simulink model "mb4k-v155"  (R2013a)
settings: Solver=VariableStepDiscrete  SolverMode=SingleTasking  StartTime=0.0  StopTime=10^4  FixedStep=1  MaxStep=1  RelTol=1e-3
undefined symbols (not defined in the file): pfb_coeff_gen_calc, sfp
libraries: xbsIndex_r4 x28825, casper_library_misc x154, casper_library_delays x126, simulink x34, dspsigops x24, xbsReplacements_r4 x20, fast_library x16, xps_library x13, casper_library_sources x2
(part 1 of 293)
settings Model: Creator=zhuyan  LastModifiedBy=zhuyan
settings Model: ModifiedComment=|
  | zhuyan -- Mon Apr 09 13:55:30 2018 -- Version 1.155
  | update_casper_blocks('mb4k')
settings Model: ModifiedHistory=|
  | zhuyan -- Mon Apr 09 13:55:30 2018 -- Version 1.155
  | update_casper_blocks('mb4k')
  | 
  | zhuyan -- Sun Apr 08 15:18:23 2018 -- Version 1.154
  | minor: change sample time of 'step_reset' and 'step_arm' block from 0 to 1
  | 
  | zhuyan -- Tue Apr 03 14:44:45 2018 -- Version 1.151
  | extend noisecal registers to 48 bits each.
  | 
  | zhuyan -- Tue Apr 03 14:44:45 2018 -- Version 1.151
  | extend noisecal registers to 48 bits each.
  | 
  | zhuyan -- Mon Apr 02 14:41:34 2018 -- Version 1.150
  | Add "pps_counter"
  | 
  | zhuyan -- Thu Mar 29 14:54:53 2018 -- Version 1.149
  | change rcs_id to "mb4k"
  | 
  | zhuyan -- Fri Mar 23 14:29:22 2018 -- Version 1.147
  | increase ADC scope from 2048 to 4096 samples each  polarization
  | 
  | zhuyan -- Fri Mar 23 14:29:22 2018 -- Version 1.147
  | 
  | increase ADC scope from 2048 to 4096 samples per polarization
  | 
  | zhuyan -- Sun Jan 14 20:12:11 2018 -- Version 1.146
  | Disable fabric at startup in tenGbE_v2 blocks
  | 
  | zhuyan -- Thu Jan 11 19:18:11 2018 -- Version 1.145
  | correct typo.
  | replace wrong "From" label "sid_raw0" connected to pkt_raw1 to "sid_raw1".
  | 
  | zhuyan -- Sat Nov 11 22:55:54 2017 -- Version 1.144
  | bugfix: bit_select bits of cross term is the same as power term
settings OptimizationCC: InlineParams=on

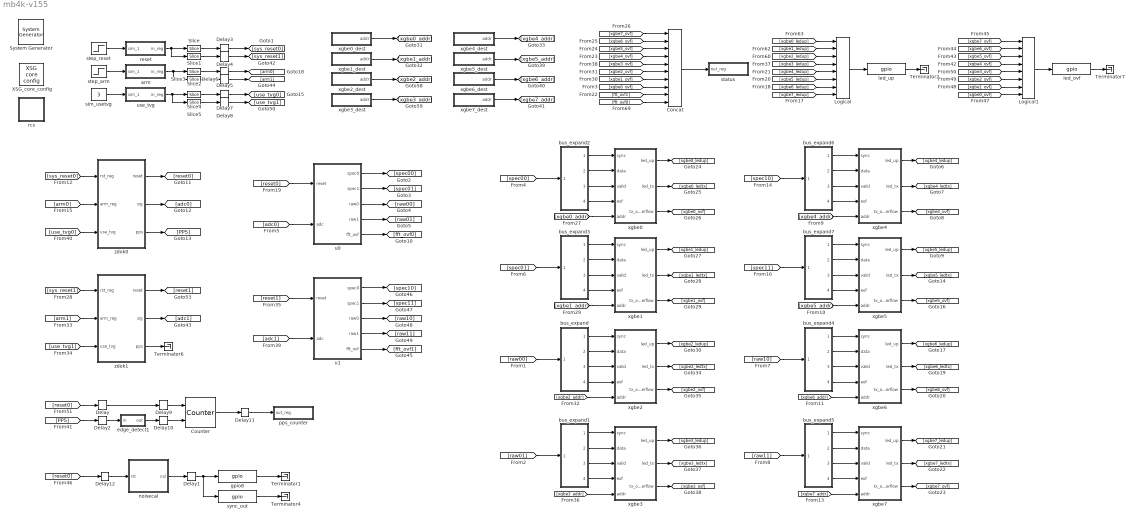
== system mb4k-v155 ==
  " System Generator" : xbsIndex_r4/ System Generator  Tag=genX  xilinxfamily=virtex6  part=xc6vsx475t  speed=-1  package=ff1759  synthesis_tool=XST  clock_wrapper=Clock Enables  directory=./mb4k/sysgen  proj_type=Project Navigator  Synth_file=XST Defaults  Impl_file=ISE Defaults  testbench=off  simulink_period=1  sysclk_period=10  dcm_input_clock_period=100  incr_netlist=off  trim_vbits=Everywhere in SubSystem  dbl_ovrd=According to Block Masks  core_generation=According to Block Masks  run_coregen=off  deprecated_control=off  eval_field=0  block_type=sysgen
  Concat : xbsIndex_r4/Concat  num_inputs=10  dbl_ovrd=off  block_type=concat
  Counter : xbsIndex_r4/Counter  cnt_type=Count Limited  cnt_to=Inf  operation=Up  start_count=0  cnt_by_val=1  arith_type=Unsigned  n_bits=32  bin_pt=0  load_pin=off  rst=on  en=on  explicit_period=on  period=1  dbl_ovrd=off  use_behavioral_HDL=on  implementation=Fabric  block_type=counter
  Delay{,1..12} (13 alike) : xbsIndex_r4/Delay  rst=off  infoeditControl=Selection of Reset will increase slice count due to use of real FFs and instead of SRLs  en=off  latency=1  dbl_ovrd=off  reg_retiming=off  block_type=delay
  Logical : xbsIndex_r4/Logical  logical_function=AND  inputs=8  en=off  latency=1  precision=Full  arith_type=Unsigned  n_bits=16  bin_pt=0  align_bp=on  dbl_ovrd=off  block_type=logical
  Logical1 : xbsIndex_r4/Logical  logical_function=OR  inputs=8  en=off  latency=1  precision=Full  arith_type=Unsigned  n_bits=16  bin_pt=0  align_bp=on  dbl_ovrd=off  block_type=logical
  Slice : xbsIndex_r4/Slice  nbits=1  boolean_output=on  mode=Lower Bit Location + Width  bit1=0  base1=MSB of Input  bit0=0  base0=LSB of Input  dbl_ovrd=off  block_type=slice
  Slice1 : xbsIndex_r4/Slice  nbits=1  boolean_output=on  mode=Lower Bit Location + Width  bit1=0  base1=MSB of Input  bit0=1  base0=LSB of Input  dbl_ovrd=off  block_type=slice
  Slice2 : xbsIndex_r4/Slice  nbits=1  boolean_output=on  mode=Lower Bit Location + Width  bit1=0  base1=MSB of Input  bit0=0  base0=LSB of Input  dbl_ovrd=off  block_type=slice
  Slice3 : xbsIndex_r4/Slice  nbits=1  boolean_output=on  mode=Lower Bit Location + Width  bit1=0  base1=MSB of Input  bit0=1  base0=LSB of Input  dbl_ovrd=off  block_type=slice
  Slice4 : xbsIndex_r4/Slice  nbits=1  boolean_output=on  mode=Lower Bit Location + Width  bit1=0  base1=MSB of Input  bit0=0  base0=LSB of Input  dbl_ovrd=off  block_type=slice
  Slice5 : xbsIndex_r4/Slice  nbits=1  boolean_output=on  mode=Lower Bit Location + Width  bit1=0  base1=MSB of Input  bit0=1  base0=LSB of Input  dbl_ovrd=off  block_type=slice
  Terminator{1..2,4,6..7} (5 alike) : Terminator
  XSG_core_config : xps_library/XSG core config  Tag=xps:xsg  hw_sys=ROACH2:sx475t  clk_src=adc0_clk  ROACH_clk_src=sys_clk  ROACH2_clk_src=adc0_clk  MKDIG_clk_src=sys_clk  clk_rate=100  sample_period=1  synthesis_tool=XST
  arm : Subsystem  Tag=xps:sw_reg  AncestorBlock=xps_library/software register  mask(swreg){io_dir=From Processor, io_delay=0, sample_period=1, names=reg, bitwidths=2, bin_pts=0, arith_types=0, sim_port=on, show_format=off}
      mask prompts: io_dir "I/O direction"; io_delay "I/O delay"; sample_period "Sample period"; names "Bitfield names [msb...lsb]"; bitwidths "Bitfield widths"; bin_pts "Bitfield binary pts"; arith_types "Bitfield types, ufix=0, fix=1, bool=2"; sim_port "Provide sim input/output?"; show_format "Print format string?"
      mask help: A 32-bit software-accessible register. Can be divided into bitfields of varying widths and types using the fields in the block mask.
      mask init:
      | swreg_init(gcb)
      settings: AttributesFormatString=1input
      mask TypeOptions io_dir: To Processor | From Processor
      mask Callback io_dir:
      | mask_enables = get_param(gcb, 'MaskEnables');
      | mask_names = get_param(gcb, 'MaskNames');
      | if strcmp(get_param(gcb, 'io_dir'), 'To Processor'),
      |     mask_enables{ismember(mask_names, 'sample_period')} = 'off';
      | else
      |     mask_enables{ismember(mask_names, 'sample_period')} = 'on';
      | end
      | set_param(gcb, 'MaskEnables', mask_enables);
  bus_expand : Subsystem  AncestorBlock=casper_library_flow_control/bus_expand  mask(bus_expand){mode=divisions of arbitrary size, outputNum=1, outputWidth=[1 64 1 1], outputBinaryPt=0, outputArithmeticType=[2 0 2 2], show_format=off, outputToWorkspace=off, variablePrefix=out, outputToModelAsWell=on}
      mask prompts: outputNum "Number of outputs:"; outputWidth "Output width:"; outputBinaryPt "Output binary point position:"; outputArithmeticType "Output arithmetic type (ufix=0, fix=1, bool=2):"; show_format "Print format string?"; outputToWorkspace "Output to workspace?"; variablePrefix "Variable name prefix:"; outputToModelAsWell "Output to model as well?"
      mask help:
      | Expands a 'bus' made using a bus_create or Xilinx
      | concat block.
      | The input will be divided into the specified number
      | of outputs with the specified arithmetic types.
      | Divisions are made from most significant bit.
      mask init:
      | bus_expand_init(gcb,...
      |     'mode', mode, ...
      |     'outputNum', outputNum, ...
      |     'outputWidth', outputWidth, ...
      |     'outputBinaryPt', outputBinaryPt, ...
      |     'outputArithmeticType', outputArithmeticType, ...
      |     'show_format', show_format, ...
      |     'outputToWorkspace', outputToWorkspace, ...
      |     'variablePrefix', variablePrefix, ...
      |     'outputToModelAsWell', outputToModelAsWell);
      settings: AttributesFormatString=4 outputs
      mask TypeOptions mode: divisions of equal size | divisions of arbitrary size
      mask Callback mode: bus_expand_callback();
      mask Callback outputWidth: bus_expand_callback();
      mask Callback outputToWorkspace: bus_expand_callback();
  bus_expand1 : Subsystem  AncestorBlock=casper_library_flow_control/bus_expand  mask(bus_expand){mode=divisions of arbitrary size, outputNum=1, outputWidth=[1 64 1 1], outputBinaryPt=0, outputArithmeticType=[2 0 2 2], show_format=off, outputToWorkspace=off, variablePrefix=out, outputToModelAsWell=on}
      mask prompts: outputNum "Number of outputs:"; outputWidth "Output width:"; outputBinaryPt "Output binary point position:"; outputArithmeticType "Output arithmetic type (ufix=0, fix=1, bool=2):"; show_format "Print format string?"; outputToWorkspace "Output to workspace?"; variablePrefix "Variable name prefix:"; outputToModelAsWell "Output to model as well?"
      mask help:
      | Expands a 'bus' made using a bus_create or Xilinx
      | concat block.
      | The input will be divided into the specified number
      | of outputs with the specified arithmetic types.
      | Divisions are made from most significant bit.
      mask init: same as mb4k-v155/bus_expand
      settings: AttributesFormatString=4 outputs
      mask TypeOptions mode: divisions of equal size | divisions of arbitrary size
      mask Callback mode: bus_expand_callback();
      mask Callback outputWidth: bus_expand_callback();
      mask Callback outputToWorkspace: bus_expand_callback();
  bus_expand2 : Subsystem  AncestorBlock=casper_library_flow_control/bus_expand  mask(bus_expand){mode=divisions of arbitrary size, outputNum=1, outputWidth=[1 64 1 1], outputBinaryPt=0, outputArithmeticType=[2 0 2 2], show_format=off, outputToWorkspace=off, variablePrefix=out, outputToModelAsWell=on}
      mask prompts: outputNum "Number of outputs:"; outputWidth "Output width:"; outputBinaryPt "Output binary point position:"; outputArithmeticType "Output arithmetic type (ufix=0, fix=1, bool=2):"; show_format "Print format string?"; outputToWorkspace "Output to workspace?"; variablePrefix "Variable name prefix:"; outputToModelAsWell "Output to model as well?"
      mask help:
      | Expands a 'bus' made using a bus_create or Xilinx
      | concat block.
      | The input will be divided into the specified number
      | of outputs with the specified arithmetic types.
      | Divisions are made from most significant bit.
      mask init: same as mb4k-v155/bus_expand
      settings: AttributesFormatString=4 outputs
      mask TypeOptions mode: divisions of equal size | divisions of arbitrary size
      mask Callback mode: bus_expand_callback();
      mask Callback outputWidth: bus_expand_callback();
      mask Callback outputToWorkspace: bus_expand_callback();
  bus_expand3 : Subsystem  AncestorBlock=casper_library_flow_control/bus_expand  mask(bus_expand){mode=divisions of arbitrary size, outputNum=1, outputWidth=[1 64 1 1], outputBinaryPt=0, outputArithmeticType=[2 0 2 2], show_format=off, outputToWorkspace=off, variablePrefix=out, outputToModelAsWell=on}
      mask prompts: outputNum "Number of outputs:"; outputWidth "Output width:"; outputBinaryPt "Output binary point position:"; outputArithmeticType "Output arithmetic type (ufix=0, fix=1, bool=2):"; show_format "Print format string?"; outputToWorkspace "Output to workspace?"; variablePrefix "Variable name prefix:"; outputToModelAsWell "Output to model as well?"
      mask help:
      | Expands a 'bus' made using a bus_create or Xilinx
      | concat block.
      | The input will be divided into the specified number
      | of outputs with the specified arithmetic types.
      | Divisions are made from most significant bit.
      mask init: same as mb4k-v155/bus_expand
      settings: AttributesFormatString=4 outputs
      mask TypeOptions mode: divisions of equal size | divisions of arbitrary size
      mask Callback mode: bus_expand_callback();
      mask Callback outputWidth: bus_expand_callback();
      mask Callback outputToWorkspace: bus_expand_callback();
  bus_expand4 : Subsystem  AncestorBlock=casper_library_flow_control/bus_expand  mask(bus_expand){mode=divisions of arbitrary size, outputNum=1, outputWidth=[1 64 1 1], outputBinaryPt=0, outputArithmeticType=[2 0 2 2], show_format=off, outputToWorkspace=off, variablePrefix=out, outputToModelAsWell=on}
      mask prompts: outputNum "Number of outputs:"; outputWidth "Output width:"; outputBinaryPt "Output binary point position:"; outputArithmeticType "Output arithmetic type (ufix=0, fix=1, bool=2):"; show_format "Print format string?"; outputToWorkspace "Output to workspace?"; variablePrefix "Variable name prefix:"; outputToModelAsWell "Output to model as well?"
      mask help:
      | Expands a 'bus' made using a bus_create or Xilinx
      | concat block.
      | The input will be divided into the specified number
      | of outputs with the specified arithmetic types.
      | Divisions are made from most significant bit.
      mask init: same as mb4k-v155/bus_expand
      settings: AttributesFormatString=4 outputs
      mask TypeOptions mode: divisions of equal size | divisions of arbitrary size
      mask Callback mode: bus_expand_callback();
      mask Callback outputWidth: bus_expand_callback();
      mask Callback outputToWorkspace: bus_expand_callback();
  bus_expand5 : Subsystem  AncestorBlock=casper_library_flow_control/bus_expand  mask(bus_expand){mode=divisions of arbitrary size, outputNum=1, outputWidth=[1 64 1 1], outputBinaryPt=0, outputArithmeticType=[2 0 2 2], show_format=off, outputToWorkspace=off, variablePrefix=out, outputToModelAsWell=on}
      mask prompts: outputNum "Number of outputs:"; outputWidth "Output width:"; outputBinaryPt "Output binary point position:"; outputArithmeticType "Output arithmetic type (ufix=0, fix=1, bool=2):"; show_format "Print format string?"; outputToWorkspace "Output to workspace?"; variablePrefix "Variable name prefix:"; outputToModelAsWell "Output to model as well?"
      mask help:
      | Expands a 'bus' made using a bus_create or Xilinx
      | concat block.
      | The input will be divided into the specified number
      | of outputs with the specified arithmetic types.
      | Divisions are made from most significant bit.
      mask init: same as mb4k-v155/bus_expand
      settings: AttributesFormatString=4 outputs
      mask TypeOptions mode: divisions of equal size | divisions of arbitrary size
      mask Callback mode: bus_expand_callback();
      mask Callback outputWidth: bus_expand_callback();
      mask Callback outputToWorkspace: bus_expand_callback();
  bus_expand6 : Subsystem  AncestorBlock=casper_library_flow_control/bus_expand  mask(bus_expand){mode=divisions of arbitrary size, outputNum=1, outputWidth=[1 64 1 1], outputBinaryPt=0, outputArithmeticType=[2 0 2 2], show_format=off, outputToWorkspace=off, variablePrefix=out, outputToModelAsWell=on}
      mask prompts: outputNum "Number of outputs:"; outputWidth "Output width:"; outputBinaryPt "Output binary point position:"; outputArithmeticType "Output arithmetic type (ufix=0, fix=1, bool=2):"; show_format "Print format string?"; outputToWorkspace "Output to workspace?"; variablePrefix "Variable name prefix:"; outputToModelAsWell "Output to model as well?"
      mask help:
      | Expands a 'bus' made using a bus_create or Xilinx
      | concat block.
      | The input will be divided into the specified number
      | of outputs with the specified arithmetic types.
      | Divisions are made from most significant bit.
      mask init: same as mb4k-v155/bus_expand
      settings: AttributesFormatString=4 outputs
      mask TypeOptions mode: divisions of equal size | divisions of arbitrary size
      mask Callback mode: bus_expand_callback();
      mask Callback outputWidth: bus_expand_callback();
      mask Callback outputToWorkspace: bus_expand_callback();
  bus_expand7 : Subsystem  AncestorBlock=casper_library_flow_control/bus_expand  mask(bus_expand){mode=divisions of arbitrary size, outputNum=1, outputWidth=[1 64 1 1], outputBinaryPt=0, outputArithmeticType=[2 0 2 2], show_format=off, outputToWorkspace=off, variablePrefix=out, outputToModelAsWell=on}
      mask prompts: outputNum "Number of outputs:"; outputWidth "Output width:"; outputBinaryPt "Output binary point position:"; outputArithmeticType "Output arithmetic type (ufix=0, fix=1, bool=2):"; show_format "Print format string?"; outputToWorkspace "Output to workspace?"; variablePrefix "Variable name prefix:"; outputToModelAsWell "Output to model as well?"
      mask help:
      | Expands a 'bus' made using a bus_create or Xilinx
      | concat block.
      | The input will be divided into the specified number
      | of outputs with the specified arithmetic types.
      | Divisions are made from most significant bit.
      mask init: same as mb4k-v155/bus_expand
      settings: AttributesFormatString=4 outputs
      mask TypeOptions mode: divisions of equal size | divisions of arbitrary size
      mask Callback mode: bus_expand_callback();
      mask Callback outputWidth: bus_expand_callback();
      mask Callback outputToWorkspace: bus_expand_callback();
  edge_detect1 : Subsystem  AncestorBlock=casper_library_misc/edge_detect  mask(edge_detect){edge=Rising, polarity=Active High, x_in=[0 0.08 0.08 0.16 0.16 0.24 0.24 0.32 0.32 0.4], y_in=[-1 -1  1  1  1  1  1  1  1  1], x_out=[0.6 0.68 0.68 0.76 0.76 0.84 0.84 0.92 0.92 1], y_out=[-1 -1  1  1 -1 -1 -1 -1 -1 -1]}
      mask prompts: edge "Edge Type"; polarity "Output Polarity"; x_in "Input X Positions"; y_in "Input Y Positions"; x_out "Output X Positions"; y_out "Output Y Positions"
      mask help: Output is active for one-cycle after each edge of the specified type (i.e. rising, falling or both).
      mask init:
      | edge_detect_init(gcb,...
      |     'edge',edge,...
      |     'polarity',polarity,...
      |     'x_in',x_in,...
      |     'x_out',x_out,...
      |     'y_in',y_in,...
      |     'y_out',y_out);
      | 
      | x_in_num=str2num(getfield(getfield(get_param(gcb,'UserData'),'parameters'),'x_in'));
      | x_out_num=str2num(getfield(getfield(get_param(gcb,'UserData'),'parameters'),'x_out'));
      | y_in_num=str2num(getfield(getfield(get_param(gcb,'UserData'),'parameters'),'y_in'));
      | y_out_num=str2num(getfield(getfield(get_param(gcb,'UserData'),'parameters'),'y_out'));
      settings: AttributesFormatString=|
      | rising edges
      | active high
      mask Help: Output is active for one-cycle after each edge of the specified type (i.e. rising, falling, or both).  Latency is zero.
      mask TypeOptions edge: Rising | Falling | Both
      mask TypeOptions polarity: Active High | Active Low
      mask Display:
      | plot(x_in_num,0.5+y_in_num*0.2);
      | plot(x_out_num,0.5+y_out_num*0.2);
  gpio8 : xps_library/gpio  Tag=xps:gpio  io_group=ROACH2:gpio  io_dir=out  arith_type=Boolean  bitwidth=1  bin_pt=0  bit_index=0  sample_period=1  use_single_ended=off  use_ddr=off  reg_iob=on  reg_clk_phase=0  termination=None
  led_ovf : xps_library/gpio  Tag=xps:gpio  io_group=ROACH2:led  io_dir=out  arith_type=Boolean  bitwidth=1  bin_pt=0  bit_index=4  sample_period=1  use_single_ended=off  use_ddr=off  reg_iob=on  reg_clk_phase=0  termination=None
  led_up : xps_library/gpio  Tag=xps:gpio  io_group=ROACH2:led  io_dir=out  arith_type=Boolean  bitwidth=1  bin_pt=0  bit_index=0  sample_period=1  use_single_ended=off  use_ddr=off  reg_iob=on  reg_clk_phase=0  termination=None
  noisecal : Subsystem  mask(noisecal){}
      mask help:
      | Square wave to control external noise source.
      |
      | delay - number of clocks before the noise on signal after reset+latency
      | delay_hipart - higher 16 bits of "delay" register, total 48 bits
      | on - number of clocks in on state
      | on_hipart - higher 16 bits of "on" register, total 48 bits
      | off -number of clocks in off state
      | off_hipart - higher 16 bits of "off" register, total 48 bits
      |
      | sequence:
      | reset->[latency]->delay->on->off->on->off->...
      mask Display:
      | port_label('input', 1, 'rst');
      | port_label('output', 1, 'cal');
      | disp('z^{-5}','texmode','on');
  pps_counter : Subsystem  Tag=xps:sw_reg  AncestorBlock=xps_library/software register  mask(swreg){io_dir=To Processor, io_delay=0, sample_period=1, names=reg, bitwidths=32, bin_pts=0, arith_types=0, sim_port=off, show_format=off}
      mask prompts: io_dir "I/O direction"; io_delay "I/O delay"; sample_period "Sample period"; names "Bitfield names [msb...lsb]"; bitwidths "Bitfield widths"; bin_pts "Bitfield binary pts"; arith_types "Bitfield types, ufix=0, fix=1, bool=2"; sim_port "Provide sim input/output?"; show_format "Print format string?"
      mask help: A 32-bit software-accessible register. Can be divided into bitfields of varying widths and types using the fields in the block mask.
      mask init:
      | swreg_init(gcb)
      settings: AttributesFormatString=1output
      mask TypeOptions io_dir: To Processor | From Processor
      mask Callback io_dir:
      | mask_enables = get_param(gcb, 'MaskEnables');
      | mask_names = get_param(gcb, 'MaskNames');
      | if strcmp(get_param(gcb, 'io_dir'), 'To Processor'),
      |     mask_enables{ismember(mask_names, 'sample_period')} = 'off';
      | else
      |     mask_enables{ismember(mask_names, 'sample_period')} = 'on';
      | end
      | set_param(gcb, 'MaskEnables', mask_enables);
  rcs : Subsystem  AncestorBlock=fast_library/myrcs  mask(myrcs){proj_id=mb4k}
      mask prompts: proj_id "Project ID:"
      mask help:
      | Revision control block.
      |
      | proj_id - exact 4 characters filled by user.
      | timestamp - seconds from unix epoch.
      | version - high 16 bits are major and low 16 bits are minor.
      |
      | The timestamp and version are taken from model properties.
      mask init:
      | blk = gcb();
      | sys = bdroot();
      | 
      | if length(proj_id) ~= 4
      |     error('Project ID must be exact 4 characters');
      | end
      | 
      | set_param(blk, 'LinkStatus', 'inactive')
      | 
      | rcs_id = proj_id(1)*256^3 + proj_id(2)*256^2 + proj_id(3)*256 + proj_id(4);
      | set_param([blk '/rcs_id'], 'const', num2str(rcs_id));
      | 
      | unix_epoch = datenum(1970, 1, 1, 0, 0, 0);
      | mdate = datenum(get_param(sys, 'LastModifiedDate'), 'ddd mmm dd HH:MM:SS yyyy');
      | unix_time = round((mdate - unix_epoch) * 24*60*60);
      | set_param([blk '/rcs_timestamp'], 'const', num2str(unix_time));
      | 
      | vers = sscanf(get_param(sys, 'ModelVersion'), '%d.%d');
      | rcs_ver = num2str(vers(1)*65536 + vers(2));
      | set_param([blk '/rcs_ver'], 'const', rcs_ver);
      mask Display: fprintf(proj_id);
  reset : Subsystem  Tag=xps:sw_reg  AncestorBlock=xps_library/software register  mask(swreg){io_dir=From Processor, io_delay=0, sample_period=1, names=reg, bitwidths=2, bin_pts=0, arith_types=0, sim_port=on, show_format=off}
      mask prompts: io_dir "I/O direction"; io_delay "I/O delay"; sample_period "Sample period"; names "Bitfield names [msb...lsb]"; bitwidths "Bitfield widths"; bin_pts "Bitfield binary pts"; arith_types "Bitfield types, ufix=0, fix=1, bool=2"; sim_port "Provide sim input/output?"; show_format "Print format string?"
      mask help: A 32-bit software-accessible register. Can be divided into bitfields of varying widths and types using the fields in the block mask.
      mask init:
      | swreg_init(gcb)
      settings: AttributesFormatString=1input
      mask TypeOptions io_dir: To Processor | From Processor
      mask Callback io_dir:
      | mask_enables = get_param(gcb, 'MaskEnables');
      | mask_names = get_param(gcb, 'MaskNames');
      | if strcmp(get_param(gcb, 'io_dir'), 'To Processor'),
      |     mask_enables{ismember(mask_names, 'sample_period')} = 'off';
      | else
      |     mask_enables{ismember(mask_names, 'sample_period')} = 'on';
      | end
      | set_param(gcb, 'MaskEnables', mask_enables);
  sim_usetvg : Constant  Value=3
  status : Subsystem  Tag=xps:sw_reg  AncestorBlock=xps_library/software register  mask(swreg){io_dir=To Processor, io_delay=0, sample_period=1, names=reg, bitwidths=12, bin_pts=0, arith_types=0, sim_port=off, show_format=off}
      mask prompts: io_dir "I/O direction"; io_delay "I/O delay"; sample_period "Sample period"; names "Bitfield names [msb...lsb]"; bitwidths "Bitfield widths"; bin_pts "Bitfield binary pts"; arith_types "Bitfield types, ufix=0, fix=1, bool=2"; sim_port "Provide sim input/output?"; show_format "Print format string?"
      mask help: A 32-bit software-accessible register. Can be divided into bitfields of varying widths and types using the fields in the block mask.
      mask init:
      | swreg_init(gcb)
      settings: AttributesFormatString=1output
      mask TypeOptions io_dir: To Processor | From Processor
      mask Callback io_dir:
      | mask_enables = get_param(gcb, 'MaskEnables');
      | mask_names = get_param(gcb, 'MaskNames');
      | if strcmp(get_param(gcb, 'io_dir'), 'To Processor'),
      |     mask_enables{ismember(mask_names, 'sample_period')} = 'off';
      | else
      |     mask_enables{ismember(mask_names, 'sample_period')} = 'on';
      | end
      | set_param(gcb, 'MaskEnables', mask_enables);
  step_arm : Step  After=7  SampleTime=1
  step_reset : Step  SampleTime=1
  sync_out : xps_library/gpio  Tag=xps:gpio  io_group=ROACH2:sync_out  io_dir=out  arith_type=Boolean  bitwidth=1  bin_pt=0  bit_index=0  sample_period=1  use_single_ended=off  use_ddr=off  reg_iob=on  reg_clk_phase=0  termination=Pulldown
  u0 : Subsystem  mask{nbins=4096}
      mask prompts: nbins "Num frequency channels:"
      mask init:
      | binbits = nextpow2(str2num(get_param(gcb, 'nbins')));
      | old_fftbits = str2num(get_param([gcb, '/x4_spec'], 'FFTSize'));
      | if binbits + 1 == old_fftbits
      |     return
      | end
      | 
      | blk = [gcb, '/x4_spec'];
      | param = 'FFTSize';
      | oldval = str2num(get_param(blk, param));
      | newval = binbits + 1;
      | if oldval ~= newval
      |     set_param(blk, param, num2str(newval));
      | end
      | 
      | blk = [gcb, '/x4_vacc'];
      | param = 'bin_bits';
      | oldval = str2num(get_param(blk, param));
      | newval = binbits;
      | if oldval ~= newval
      |     set_param(blk, param, num2str(newval));
      | end
      | oldval = get_param(blk, param);
      | 
      | blk = [gcb, '/pkt_vacc0'];
      | param = 'nbits';
      | oldval = str2num(get_param(blk, param));
      | newval = binbits - 1;
      | if newval > 10
      |     newval = 10;
      | end
      | if oldval ~= newval
      |     set_param(blk, param, num2str(newval));
      | end
      | 
      | blk = [gcb, '/pkt_vacc1'];
      | param = 'nbits';
      | oldval = str2num(get_param(blk, param));
      | if oldval ~= newval
      |     set_param(blk, param, num2str(newval));
      | end
      mask TypeOptions nbins: 1024 | 2048 | 4096 | 8192
  u1 : Subsystem  mask{nbins=4096}
      mask prompts: nbins "Num frequency channels:"
      mask init: same as mb4k-v155/u0
      mask TypeOptions nbins: 1024 | 2048 | 4096 | 8192
  use_tvg : Subsystem  Tag=xps:sw_reg  AncestorBlock=xps_library/software register  mask(swreg){io_dir=From Processor, io_delay=0, sample_period=1, names=reg, bitwidths=2, bin_pts=0, arith_types=0, sim_port=on, show_format=off}
      mask prompts: io_dir "I/O direction"; io_delay "I/O delay"; sample_period "Sample period"; names "Bitfield names [msb...lsb]"; bitwidths "Bitfield widths"; bin_pts "Bitfield binary pts"; arith_types "Bitfield types, ufix=0, fix=1, bool=2"; sim_port "Provide sim input/output?"; show_format "Print format string?"
      mask help: A 32-bit software-accessible register. Can be divided into bitfields of varying widths and types using the fields in the block mask.
      mask init:
      | swreg_init(gcb)
      settings: AttributesFormatString=1input
      mask TypeOptions io_dir: To Processor | From Processor
      mask Callback io_dir:
      | mask_enables = get_param(gcb, 'MaskEnables');
      | mask_names = get_param(gcb, 'MaskNames');
      | if strcmp(get_param(gcb, 'io_dir'), 'To Processor'),
      |     mask_enables{ismember(mask_names, 'sample_period')} = 'off';
      | else
      |     mask_enables{ismember(mask_names, 'sample_period')} = 'on';
      | end
      | set_param(gcb, 'MaskEnables', mask_enables);
  xgbe0 : Subsystem
  xgbe0_dest : Subsystem
  xgbe1 : Subsystem
  xgbe1_dest : Subsystem
  xgbe2 : Subsystem
  xgbe2_dest : Subsystem
  xgbe3 : Subsystem
  xgbe3_dest : Subsystem
  xgbe4 : Subsystem
  xgbe4_dest : Subsystem
  xgbe5 : Subsystem
  xgbe5_dest : Subsystem
  xgbe6 : Subsystem
  xgbe6_dest : Subsystem
  xgbe7 : Subsystem
  xgbe7_dest : Subsystem
  zdok0 : Subsystem  mask{adc_clk_rate=1024}
      mask prompts: adc_clk_rate "ADC clock rate (MHz)"
  zdok1 : Subsystem  mask{adc_clk_rate=1024}
      mask prompts: adc_clk_rate "ADC clock rate (MHz)"
  wires:
  step_reset/1 -> reset/1(sim_1)
  reset/1(in_reg) -> Slice1/1, Slice/1
  sim_usetvg/1 -> use_tvg/1(sim_1)
  use_tvg/1(in_reg) -> Slice4/1, Slice5/1
  step_arm/1 -> arm/1(sim_1)
  arm/1(in_reg) -> Slice3/1, Slice2/1
  [use_tvg0] -> zdok0/3(use_tvg)
  [arm0] -> zdok0/2(arm_reg)
  [sys_reset0] -> zdok0/1(rst_reg)
  zdok0/3(pps) -> [PPS]
  zdok0/1(reset) -> [reset0]
  zdok0/2(sig) -> [adc0]
  xgbe0/1(led_up) -> [xgbe0_ledup]
  xgbe0/2(led_tx) -> [xgbe0_ledtx]
  xgbe0/3(tx_overflow) -> [xgbe0_ovf]
  xgbe1/1(led_up) -> [xgbe1_ledup]
  xgbe1/2(led_tx) -> [xgbe1_ledtx]
  xgbe1/3(tx_overflow) -> [xgbe1_ovf]
  xgbe0_dest/1(addr) -> [xgbe0_addr]
  xgbe1_dest/1(addr) -> [xgbe1_addr]
  [xgbe0_addr] -> xgbe0/5(addr)
  [xgbe1_addr] -> xgbe1/5(addr)
  Logical/1 -> led_up/1
  led_up/1 -> Terminator2/1
  Concat/1 -> status/1(out_reg)
  bus_expand/1(msb_out4) -> xgbe2/1(sync)
  bus_expand/2(out3) -> xgbe2/2(data)
  bus_expand/3(out2) -> xgbe2/3(valid)
  bus_expand/4(lsb_out1) -> xgbe2/4(eof)
  [xgbe2_addr] -> xgbe2/5(addr)
  xgbe2/1(led_up) -> [xgbe2_ledup]
  xgbe2/2(led_tx) -> [xgbe2_ledtx]
  xgbe2/3(tx_overflow) -> [xgbe2_ovf]
  [xgbe3_addr] -> xgbe3/5(addr)
  xgbe3/1(led_up) -> [xgbe3_ledup]
  xgbe3/2(led_tx) -> [xgbe3_ledtx]
  xgbe3/3(tx_overflow) -> [xgbe3_ovf]
  xgbe2_dest/1(addr) -> [xgbe2_addr]
  xgbe3_dest/1(addr) -> [xgbe3_addr]
  [xgbe1_ledup] -> Logical/2
  [xgbe2_ledup] -> Logical/3
  [xgbe0_ledup] -> Logical/1
  [xgbe3_ledup] -> Logical/4
  bus_expand2/1(msb_out4) -> xgbe0/1(sync)
  bus_expand3/1(msb_out4) -> xgbe1/1(sync)
  bus_expand2/2(out3) -> xgbe0/2(data)
  bus_expand2/3(out2) -> xgbe0/3(valid)
  bus_expand2/4(lsb_out1) -> xgbe0/4(eof)
  bus_expand3/3(out2) -> xgbe1/3(valid)
  bus_expand3/4(lsb_out1) -> xgbe1/4(eof)
  bus_expand3/2(out3) -> xgbe1/2(data)
  [reset0] -> u0/1(reset)
  [adc0] -> u0/2(adc)
  u0/1(spec0) -> [spec00]
  [raw00] -> bus_expand/1(bus_in)
  [raw01] -> bus_expand1/1(bus_in)
  bus_expand1/1(msb_out4) -> xgbe3/1(sync)
  bus_expand1/2(out3) -> xgbe3/2(data)
  bus_expand1/3(out2) -> xgbe3/3(valid)
  bus_expand1/4(lsb_out1) -> xgbe3/4(eof)
  [spec00] -> bus_expand2/1(bus_in)
  [spec01] -> bus_expand3/1(bus_in)
  u0/2(spec1) -> [spec01]
  u0/3(raw0) -> [raw00]
  u0/4(raw1) -> [raw01]
  u0/5(fft_ovf) -> [fft_ovf0]
  xgbe4/1(led_up) -> [xgbe4_ledup]
  xgbe4/2(led_tx) -> [xgbe4_ledtx]
  xgbe4/3(tx_overflow) -> [xgbe4_ovf]
  xgbe5/1(led_up) -> [xgbe5_ledup]
  xgbe5/2(led_tx) -> [xgbe5_ledtx]
  xgbe5/3(tx_overflow) -> [xgbe5_ovf]
  [xgbe4_addr] -> xgbe4/5(addr)
  [xgbe5_addr] -> xgbe5/5(addr)
  bus_expand4/1(msb_out4) -> xgbe6/1(sync)
  bus_expand4/2(out3) -> xgbe6/2(data)
  bus_expand4/3(out2) -> xgbe6/3(valid)
  bus_expand4/4(lsb_out1) -> xgbe6/4(eof)
  [xgbe6_addr] -> xgbe6/5(addr)
  xgbe6/1(led_up) -> [xgbe6_ledup]
  xgbe6/2(led_tx) -> [xgbe6_ledtx]
  xgbe6/3(tx_overflow) -> [xgbe6_ovf]
  [xgbe7_addr] -> xgbe7/5(addr)
  xgbe7/1(led_up) -> [xgbe7_ledup]
  xgbe7/2(led_tx) -> [xgbe7_ledtx]
  xgbe7/3(tx_overflow) -> [xgbe7_ovf]
  bus_expand6/1(msb_out4) -> xgbe4/1(sync)
  bus_expand7/1(msb_out4) -> xgbe5/1(sync)
  bus_expand6/2(out3) -> xgbe4/2(data)
  bus_expand6/3(out2) -> xgbe4/3(valid)
  bus_expand6/4(lsb_out1) -> xgbe4/4(eof)
  bus_expand7/3(out2) -> xgbe5/3(valid)
  bus_expand7/4(lsb_out1) -> xgbe5/4(eof)
  bus_expand7/2(out3) -> xgbe5/2(data)
  [raw10] -> bus_expand4/1(bus_in)
  [raw11] -> bus_expand5/1(bus_in)
  bus_expand5/1(msb_out4) -> xgbe7/1(sync)
  bus_expand5/2(out3) -> xgbe7/2(data)
  bus_expand5/3(out2) -> xgbe7/3(valid)
  bus_expand5/4(lsb_out1) -> xgbe7/4(eof)
  [spec10] -> bus_expand6/1(bus_in)
  [spec11] -> bus_expand7/1(bus_in)
  xgbe4_dest/1(addr) -> [xgbe4_addr]
  xgbe5_dest/1(addr) -> [xgbe5_addr]
  xgbe6_dest/1(addr) -> [xgbe6_addr]
  xgbe7_dest/1(addr) -> [xgbe7_addr]
  [xgbe6_ledup] -> Logical/7
  [xgbe5_ledup] -> Logical/6
  [xgbe4_ledup] -> Logical/5
  [xgbe7_ledup] -> Logical/8
  [use_tvg1] -> zdok1/3(use_tvg)
  [arm1] -> zdok1/2(arm_reg)
  [sys_reset1] -> zdok1/1(rst_reg)
  zdok1/3(pps) -> Terminator6/1
  zdok1/1(reset) -> [reset1]
  zdok1/2(sig) -> [adc1]
  [reset1] -> u1/1(reset)
  [adc1] -> u1/2(adc)
  u1/1(spec0) -> [spec10]
  u1/2(spec1) -> [spec11]
  u1/3(raw0) -> [raw10]
  u1/4(raw1) -> [raw11]
  u1/5(fft_ovf) -> [fft_ovf1]
  [reset0] -> Delay12/1
  noisecal/1(cal) -> Delay1/1
  gpio8/1 -> Terminator1/1
  Delay1/1 -> gpio8/1, sync_out/1
  sync_out/1 -> Terminator4/1
  Logical1/1 -> led_ovf/1
  led_ovf/1 -> Terminator7/1
  [xgbe7_ovf] -> Logical1/1
  [xgbe0_ovf] -> Logical1/8
  [xgbe4_ovf] -> Logical1/4
  [xgbe5_ovf] -> Logical1/3
  [xgbe6_ovf] -> Logical1/2
  [xgbe1_ovf] -> Logical1/7
  [xgbe3_ovf] -> Logical1/5
  [xgbe2_ovf] -> Logical1/6
  Slice/1 -> Delay3/1
  Slice1/1 -> Delay4/1
  Slice2/1 -> Delay5/1
  Slice3/1 -> Delay6/1
  Slice4/1 -> Delay7/1
  Slice5/1 -> Delay8/1
  Delay3/1 -> [sys_reset0]
  Delay4/1 -> [sys_reset1]
  Delay5/1 -> [arm0]
  Delay6/1 -> [arm1]
  Delay7/1 -> [use_tvg0]
  Delay8/1 -> [use_tvg1]
  [xgbe5_ovf] -> Concat/3
  [xgbe7_ovf] -> Concat/1
  [xgbe4_ovf] -> Concat/4
  [xgbe3_ovf] -> Concat/5
  [xgbe1_ovf] -> Concat/7
  [xgbe6_ovf] -> Concat/2
  [fft_ovf1] -> Concat/9
  [xgbe0_ovf] -> Concat/8
  [xgbe2_ovf] -> Concat/6
  [fft_ovf0] -> Concat/10
  [PPS] -> Delay2/1
  [reset0] -> Delay/1
  Delay/1 -> Delay9/1
  Delay2/1 -> edge_detect1/1(in)
  edge_detect1/1(out) -> Delay10/1
  Delay9/1 -> Counter/1
  Delay10/1 -> Counter/2
  Counter/1 -> Delay11/1
  Delay11/1 -> pps_counter/1(out_reg)
  Delay12/1 -> noisecal/1(rst)

== system mb4k-v155/arm ==
  sim_1 : Inport
  io_delay : xbsIndex_r4/Delay  rst=off  infoeditControl=Selection of Reset will increase slice count due to use of real FFs and instead of SRLs  en=off  latency=0  dbl_ovrd=off  reg_retiming=on  block_type=delay
  mb4k_arm_user_data_out : xbsIndex_r4/Gateway In  gui_display_data_type=Fixed-point  arith_type=Unsigned  n_bits=32  bin_pt=0  preci_type=Single  exp_width=8  frac_width=24  quantization="Round  (unbiased: +/- Inf)"  overflow=Saturate  period=1  dbl_ovrd=off  timing_constraint=None  locs_specified=off  LOCs={}  UseAsADC=off  ADCChannel='1'  inherit_from_input=off  hdl_port=on  block_type=gatewayin
  reint1 : xbsIndex_r4/Reinterpret  force_arith_type=on  arith_type=Unsigned  force_bin_pt=on  bin_pt=0  block_type=reinterpret
  slice_reg : xbsIndex_r4/Slice  nbits=2  boolean_output=off  mode=Lower Bit Location + Width  bit1=0  base1=MSB of Input  bit0=0  base0=LSB of Input  dbl_ovrd=off  block_type=slice
  in_reg : Outport
  wires:
  mb4k_arm_user_data_out/1 -> io_delay/1
  sim_1/1 -> mb4k_arm_user_data_out/1
  io_delay/1 -> slice_reg/1
  slice_reg/1 -> reint1/1
  reint1/1 -> in_reg/1

== system mb4k-v155/bus_expand ==
  bus_in : Inport
  reinterpret3 : xbsIndex_r4/Reinterpret  force_arith_type=on  arith_type=Unsigned  force_bin_pt=on  bin_pt=0  block_type=reinterpret
  slice1 : xbsIndex_r4/Slice  nbits=1  boolean_output=on  mode=Upper Bit Location + Width  bit1=-66  base1=MSB of Input  bit0=0  base0=LSB of Input  dbl_ovrd=off  block_type=slice
  slice2 : xbsIndex_r4/Slice  nbits=1  boolean_output=on  mode=Upper Bit Location + Width  bit1=-65  base1=MSB of Input  bit0=0  base0=LSB of Input  dbl_ovrd=off  block_type=slice
  slice3 : xbsIndex_r4/Slice  nbits=64  boolean_output=off  mode=Upper Bit Location + Width  bit1=-1  base1=MSB of Input  bit0=0  base0=LSB of Input  dbl_ovrd=off  block_type=slice
  slice4 : xbsIndex_r4/Slice  nbits=1  boolean_output=on  mode=Upper Bit Location + Width  bit1=0  base1=MSB of Input  bit0=0  base0=LSB of Input  dbl_ovrd=off  block_type=slice
  msb_out4 : Outport
  out3 : Outport  Port=2
  out2 : Outport  Port=3
  lsb_out1 : Outport  Port=4
  wires:
  bus_in/1 -> slice4/1, slice3/1, slice2/1, slice1/1
  slice4/1 -> msb_out4/1
  slice3/1 -> reinterpret3/1
  reinterpret3/1 -> out3/1
  slice2/1 -> out2/1
  slice1/1 -> lsb_out1/1

== system mb4k-v155/bus_expand1 ==
  bus_in : Inport
  reinterpret3 : xbsIndex_r4/Reinterpret  force_arith_type=on  arith_type=Unsigned  force_bin_pt=on  bin_pt=0  block_type=reinterpret
  slice1 : xbsIndex_r4/Slice  nbits=1  boolean_output=on  mode=Upper Bit Location + Width  bit1=-66  base1=MSB of Input  bit0=0  base0=LSB of Input  dbl_ovrd=off  block_type=slice
  slice2 : xbsIndex_r4/Slice  nbits=1  boolean_output=on  mode=Upper Bit Location + Width  bit1=-65  base1=MSB of Input  bit0=0  base0=LSB of Input  dbl_ovrd=off  block_type=slice
  slice3 : xbsIndex_r4/Slice  nbits=64  boolean_output=off  mode=Upper Bit Location + Width  bit1=-1  base1=MSB of Input  bit0=0  base0=LSB of Input  dbl_ovrd=off  block_type=slice
  slice4 : xbsIndex_r4/Slice  nbits=1  boolean_output=on  mode=Upper Bit Location + Width  bit1=0  base1=MSB of Input  bit0=0  base0=LSB of Input  dbl_ovrd=off  block_type=slice
  msb_out4 : Outport
  out3 : Outport  Port=2
  out2 : Outport  Port=3
  lsb_out1 : Outport  Port=4
  wires:
  bus_in/1 -> slice4/1, slice3/1, slice2/1, slice1/1
  slice4/1 -> msb_out4/1
  slice3/1 -> reinterpret3/1
  reinterpret3/1 -> out3/1
  slice2/1 -> out2/1
  slice1/1 -> lsb_out1/1

== system mb4k-v155/bus_expand2 ==
  bus_in : Inport
  reinterpret3 : xbsIndex_r4/Reinterpret  force_arith_type=on  arith_type=Unsigned  force_bin_pt=on  bin_pt=0  block_type=reinterpret
  slice1 : xbsIndex_r4/Slice  nbits=1  boolean_output=on  mode=Upper Bit Location + Width  bit1=-66  base1=MSB of Input  bit0=0  base0=LSB of Input  dbl_ovrd=off  block_type=slice
  slice2 : xbsIndex_r4/Slice  nbits=1  boolean_output=on  mode=Upper Bit Location + Width  bit1=-65  base1=MSB of Input  bit0=0  base0=LSB of Input  dbl_ovrd=off  block_type=slice
  slice3 : xbsIndex_r4/Slice  nbits=64  boolean_output=off  mode=Upper Bit Location + Width  bit1=-1  base1=MSB of Input  bit0=0  base0=LSB of Input  dbl_ovrd=off  block_type=slice
  slice4 : xbsIndex_r4/Slice  nbits=1  boolean_output=on  mode=Upper Bit Location + Width  bit1=0  base1=MSB of Input  bit0=0  base0=LSB of Input  dbl_ovrd=off  block_type=slice
  msb_out4 : Outport
  out3 : Outport  Port=2
  out2 : Outport  Port=3
  lsb_out1 : Outport  Port=4
  wires:
  bus_in/1 -> slice4/1, slice3/1, slice2/1, slice1/1
  slice4/1 -> msb_out4/1
  slice3/1 -> reinterpret3/1
  reinterpret3/1 -> out3/1
  slice2/1 -> out2/1
  slice1/1 -> lsb_out1/1

== system mb4k-v155/bus_expand3 ==
  bus_in : Inport
  reinterpret3 : xbsIndex_r4/Reinterpret  force_arith_type=on  arith_type=Unsigned  force_bin_pt=on  bin_pt=0  block_type=reinterpret
  slice1 : xbsIndex_r4/Slice  nbits=1  boolean_output=on  mode=Upper Bit Location + Width  bit1=-66  base1=MSB of Input  bit0=0  base0=LSB of Input  dbl_ovrd=off  block_type=slice
  slice2 : xbsIndex_r4/Slice  nbits=1  boolean_output=on  mode=Upper Bit Location + Width  bit1=-65  base1=MSB of Input  bit0=0  base0=LSB of Input  dbl_ovrd=off  block_type=slice
  slice3 : xbsIndex_r4/Slice  nbits=64  boolean_output=off  mode=Upper Bit Location + Width  bit1=-1  base1=MSB of Input  bit0=0  base0=LSB of Input  dbl_ovrd=off  block_type=slice
  slice4 : xbsIndex_r4/Slice  nbits=1  boolean_output=on  mode=Upper Bit Location + Width  bit1=0  base1=MSB of Input  bit0=0  base0=LSB of Input  dbl_ovrd=off  block_type=slice
  msb_out4 : Outport
  out3 : Outport  Port=2
  out2 : Outport  Port=3
  lsb_out1 : Outport  Port=4
  wires:
  bus_in/1 -> slice4/1, slice3/1, slice2/1, slice1/1
  slice4/1 -> msb_out4/1
  slice3/1 -> reinterpret3/1
  reinterpret3/1 -> out3/1
  slice2/1 -> out2/1
  slice1/1 -> lsb_out1/1

== system mb4k-v155/bus_expand4 ==
  bus_in : Inport
  reinterpret3 : xbsIndex_r4/Reinterpret  force_arith_type=on  arith_type=Unsigned  force_bin_pt=on  bin_pt=0  block_type=reinterpret
  slice1 : xbsIndex_r4/Slice  nbits=1  boolean_output=on  mode=Upper Bit Location + Width  bit1=-66  base1=MSB of Input  bit0=0  base0=LSB of Input  dbl_ovrd=off  block_type=slice
  slice2 : xbsIndex_r4/Slice  nbits=1  boolean_output=on  mode=Upper Bit Location + Width  bit1=-65  base1=MSB of Input  bit0=0  base0=LSB of Input  dbl_ovrd=off  block_type=slice
  slice3 : xbsIndex_r4/Slice  nbits=64  boolean_output=off  mode=Upper Bit Location + Width  bit1=-1  base1=MSB of Input  bit0=0  base0=LSB of Input  dbl_ovrd=off  block_type=slice
  slice4 : xbsIndex_r4/Slice  nbits=1  boolean_output=on  mode=Upper Bit Location + Width  bit1=0  base1=MSB of Input  bit0=0  base0=LSB of Input  dbl_ovrd=off  block_type=slice
  msb_out4 : Outport
  out3 : Outport  Port=2
  out2 : Outport  Port=3
  lsb_out1 : Outport  Port=4
  wires:
  bus_in/1 -> slice4/1, slice3/1, slice2/1, slice1/1
  slice4/1 -> msb_out4/1
  slice3/1 -> reinterpret3/1
  reinterpret3/1 -> out3/1
  slice2/1 -> out2/1
  slice1/1 -> lsb_out1/1

== system mb4k-v155/bus_expand5 ==
  bus_in : Inport
  reinterpret3 : xbsIndex_r4/Reinterpret  force_arith_type=on  arith_type=Unsigned  force_bin_pt=on  bin_pt=0  block_type=reinterpret
  slice1 : xbsIndex_r4/Slice  nbits=1  boolean_output=on  mode=Upper Bit Location + Width  bit1=-66  base1=MSB of Input  bit0=0  base0=LSB of Input  dbl_ovrd=off  block_type=slice
  slice2 : xbsIndex_r4/Slice  nbits=1  boolean_output=on  mode=Upper Bit Location + Width  bit1=-65  base1=MSB of Input  bit0=0  base0=LSB of Input  dbl_ovrd=off  block_type=slice
  slice3 : xbsIndex_r4/Slice  nbits=64  boolean_output=off  mode=Upper Bit Location + Width  bit1=-1  base1=MSB of Input  bit0=0  base0=LSB of Input  dbl_ovrd=off  block_type=slice
  slice4 : xbsIndex_r4/Slice  nbits=1  boolean_output=on  mode=Upper Bit Location + Width  bit1=0  base1=MSB of Input  bit0=0  base0=LSB of Input  dbl_ovrd=off  block_type=slice
  msb_out4 : Outport
  out3 : Outport  Port=2
  out2 : Outport  Port=3
  lsb_out1 : Outport  Port=4
  wires:
  bus_in/1 -> slice4/1, slice3/1, slice2/1, slice1/1
  slice4/1 -> msb_out4/1
  slice3/1 -> reinterpret3/1
  reinterpret3/1 -> out3/1
  slice2/1 -> out2/1
  slice1/1 -> lsb_out1/1

== system mb4k-v155/bus_expand6 ==
  bus_in : Inport
  reinterpret3 : xbsIndex_r4/Reinterpret  force_arith_type=on  arith_type=Unsigned  force_bin_pt=on  bin_pt=0  block_type=reinterpret
  slice1 : xbsIndex_r4/Slice  nbits=1  boolean_output=on  mode=Upper Bit Location + Width  bit1=-66  base1=MSB of Input  bit0=0  base0=LSB of Input  dbl_ovrd=off  block_type=slice
  slice2 : xbsIndex_r4/Slice  nbits=1  boolean_output=on  mode=Upper Bit Location + Width  bit1=-65  base1=MSB of Input  bit0=0  base0=LSB of Input  dbl_ovrd=off  block_type=slice
  slice3 : xbsIndex_r4/Slice  nbits=64  boolean_output=off  mode=Upper Bit Location + Width  bit1=-1  base1=MSB of Input  bit0=0  base0=LSB of Input  dbl_ovrd=off  block_type=slice
  slice4 : xbsIndex_r4/Slice  nbits=1  boolean_output=on  mode=Upper Bit Location + Width  bit1=0  base1=MSB of Input  bit0=0  base0=LSB of Input  dbl_ovrd=off  block_type=slice
  msb_out4 : Outport
  out3 : Outport  Port=2
  out2 : Outport  Port=3
  lsb_out1 : Outport  Port=4
  wires:
  bus_in/1 -> slice4/1, slice3/1, slice2/1, slice1/1
  slice4/1 -> msb_out4/1
  slice3/1 -> reinterpret3/1
  reinterpret3/1 -> out3/1
  slice2/1 -> out2/1
  slice1/1 -> lsb_out1/1

== system mb4k-v155/bus_expand7 ==
  bus_in : Inport
  reinterpret3 : xbsIndex_r4/Reinterpret  force_arith_type=on  arith_type=Unsigned  force_bin_pt=on  bin_pt=0  block_type=reinterpret
  slice1 : xbsIndex_r4/Slice  nbits=1  boolean_output=on  mode=Upper Bit Location + Width  bit1=-66  base1=MSB of Input  bit0=0  base0=LSB of Input  dbl_ovrd=off  block_type=slice
  slice2 : xbsIndex_r4/Slice  nbits=1  boolean_output=on  mode=Upper Bit Location + Width  bit1=-65  base1=MSB of Input  bit0=0  base0=LSB of Input  dbl_ovrd=off  block_type=slice
  slice3 : xbsIndex_r4/Slice  nbits=64  boolean_output=off  mode=Upper Bit Location + Width  bit1=-1  base1=MSB of Input  bit0=0  base0=LSB of Input  dbl_ovrd=off  block_type=slice
  slice4 : xbsIndex_r4/Slice  nbits=1  boolean_output=on  mode=Upper Bit Location + Width  bit1=0  base1=MSB of Input  bit0=0  base0=LSB of Input  dbl_ovrd=off  block_type=slice
  msb_out4 : Outport
  out3 : Outport  Port=2
  out2 : Outport  Port=3
  lsb_out1 : Outport  Port=4
  wires:
  bus_in/1 -> slice4/1, slice3/1, slice2/1, slice1/1
  slice4/1 -> msb_out4/1
  slice3/1 -> reinterpret3/1
  reinterpret3/1 -> out3/1
  slice2/1 -> out2/1
  slice1/1 -> lsb_out1/1

== system mb4k-v155/edge_detect1 ==
  in : Inport
  Delay : xbsIndex_r4/Delay  rst=off  infoeditControl=Selection of Reset will increase slice count due to use of real FFs and instead of SRLs  en=off  latency=1  dbl_ovrd=off  reg_retiming=off  block_type=delay
      settings: xl_area=[0, 0, 0, 0, 0, 0, 0]
  Inverter : xbsIndex_r4/Inverter  en=off  latency=0  dbl_ovrd=off  block_type=inv
      settings: xl_area=[0, 0, 0, 0, 0, 0, 0]
  edge_op : xbsIndex_r4/Logical  logical_function=NOR  inputs=2  en=off  latency=0  precision=Full  arith_type=Unsigned  n_bits=8  bin_pt=2  align_bp=on  dbl_ovrd=off  block_type=logical
      settings: xl_area=[0, 0, 0, 0, 0, 0, 0]
  out : Outport
  wires:
  Inverter/1 -> edge_op/1
  edge_op/1 -> out/1
  in/1 -> Inverter/1, Delay/1
  Delay/1 -> edge_op/2
